simulink library "lib_all"  (Simulink 7.6)
settings: Solver=ode45  SolverMode=SingleTasking  StartTime=0.0  StopTime=10.0  RelTol=1e-3
values from neighbouring files (symbols this model uses):
  map_lib_03 = [6.8679 7.0159 7.4571 8.1849 9.1892 10.456 11.97 13.71 15.655 17.781 20.063 22.474 ... (803 elements, 73x11)]    [scr_simdata.mat]    (also in scr_simdata_all.mat)
  map_lib_06 = [2.5784 2.6727 2.9588 3.4453 4.1444 5.068 6.226 7.6242 9.2634 11.139 13.24 15.552 ... (803 elements, 73x11)]    [scr_simdata.mat]    (also in scr_simdata_all.mat)
  map_lib_09 = [0.0025784 0.0026727 0.0029588 0.0034453 0.0041444 0.005068 0.006226 0.0076242 0.0092634 0.011139 0.01324 0.015552 ... (803 elements, 73x11)]    [scr_simdata.mat]    (also in scr_simdata_all.mat)
  map_lib_10 = [2.74 2.75 2.76 2.77 2.78 2.79 2.8 2.81 2.82 2.83 2.84 2.85 ... (81 elements, 1x81)]    [scr_simdata.mat]    (also in scr_simdata_all.mat)
  map_lib_11 = [1.1983 1.2027 1.207 1.2113 1.2156 1.2198 1.2241 1.2283 1.2326 1.2368 1.241 1.2451 ... (81 elements, 1x81)]    [scr_simdata.mat]    (also in scr_simdata_all.mat)
  map_lib_12 = [2.74 2.75 2.76 2.77 2.78 2.79 2.8 2.81 2.82 2.83 2.84 2.85 ... (81 elements, 1x81)]    [scr_simdata.mat]    (also in scr_simdata_all.mat)
  map_lib_13 = [1.74 1.75 1.76 1.77 1.78 1.79 1.8 1.81 1.82 1.83 1.84 1.85 ... (81 elements, 1x81)]    [scr_simdata.mat]    (also in scr_simdata_all.mat)
  map_lib_16 = [0.00048828125 1.6159 1.8962 2.0821 2.2251 2.3426 2.4433 2.5318 2.611 2.683 2.749 2.8101 ... (1419 elements, 129x11)]    [scr_simdata.mat]    (also in scr_simdata_all.mat)
  map_mr2_01 = [0 800 1600 2400 3200 4000]    [scr_simdata.mat]    (also in scr_simdata_all.mat)
  map_mr2_02 = [1.32 1.32 1.33 1.34 1.36 1.38]    [scr_simdata.mat]    (also in scr_simdata_all.mat)
  map_mr2_03 = [0 800 1600 2400 3200 4000]    [scr_simdata.mat]    (also in scr_simdata_all.mat)
  map_mr2_04 = [1.32 1.32 1.33 1.34 1.36 1.38]    [scr_simdata.mat]    (also in scr_simdata_all.mat)
  map_mr2_05 = [0 800 1600 2400 3200 4000]    [scr_simdata.mat]    (also in scr_simdata_all.mat)
  map_mr2_06 = [1.32 1.32 1.33 1.34 1.36 1.38]    [scr_simdata.mat]    (also in scr_simdata_all.mat)
  Parameter1 = 6    [scr_simdata.mat]    (also in scr_simdata_all.mat)
  Parameter101 = 30    [scr_simdata.mat]    (also in scr_simdata_all.mat)
  Parameter102 = 12    [scr_simdata.mat]    (also in scr_simdata_all.mat)
  Parameter103 = -240    [scr_simdata.mat]    (also in scr_simdata_all.mat)
  Parameter108 = 48    [scr_simdata.mat]    (also in scr_simdata_all.mat)
  Parameter120 = 16    [scr_simdata.mat]    (also in scr_simdata_all.mat)
  Parameter121 = 87    [scr_simdata.mat]    (also in scr_simdata_all.mat)
  Parameter123 = 78    [scr_simdata.mat]    (also in scr_simdata_all.mat)
  Parameter124 = 60    [scr_simdata.mat]    (also in scr_simdata_all.mat)
  Parameter125 = -480    [scr_simdata.mat]    (also in scr_simdata_all.mat)
  Parameter130 = 96    [scr_simdata.mat]    (also in scr_simdata_all.mat)
  Parameter131 = 3    [scr_simdata.mat]    (also in scr_simdata_all.mat)
  Parameter144 = 3    [scr_simdata.mat]    (also in scr_simdata_all.mat)
  Parameter18 = 128    [scr_simdata.mat]    (also in scr_simdata_all.mat)
  Parameter19 = 16    [scr_simdata.mat]    (also in scr_simdata_all.mat)
  Parameter2 = 12    [scr_simdata.mat]    (also in scr_simdata_all.mat)
  Parameter20 = 7    [scr_simdata.mat]    (also in scr_simdata_all.mat)
  Parameter21 = 4    [scr_simdata.mat]    (also in scr_simdata_all.mat)
  Parameter3 = 18    [scr_simdata.mat]    (also in scr_simdata_all.mat)
  Parameter32 = 1    [scr_simdata.mat]    (also in scr_simdata_all.mat)
  Parameter34 = 24    [scr_simdata.mat]    (also in scr_simdata_all.mat)
  Parameter4 = 138    [scr_simdata.mat]    (also in scr_simdata_all.mat)
  Parameter42 = 48    [scr_simdata.mat]    (also in scr_simdata_all.mat)
  Parameter5 = 135    [scr_simdata.mat]    (also in scr_simdata_all.mat)
  Parameter55 = 0    [scr_simdata.mat]    (also in scr_simdata_all.mat)
  Parameter56 = 0    [scr_simdata.mat]    (also in scr_simdata_all.mat)
  Parameter57 = 1    [scr_simdata.mat]    (also in scr_simdata_all.mat)
  Parameter58 = 1    [scr_simdata.mat]    (also in scr_simdata_all.mat)
  Parameter59 = 2    [scr_simdata.mat]    (also in scr_simdata_all.mat)
  Parameter6 = 132    [scr_simdata.mat]    (also in scr_simdata_all.mat)
  Parameter60 = 2    [scr_simdata.mat]    (also in scr_simdata_all.mat)
  Parameter63 = 144    [scr_simdata.mat]    (also in scr_simdata_all.mat)
  Parameter7 = 126    [scr_simdata.mat]    (also in scr_simdata_all.mat)
  Parameter76 = 64    [scr_simdata.mat]    (also in scr_simdata_all.mat)
  Parameter77 = 135    [scr_simdata.mat]    (also in scr_simdata_all.mat)
  Parameter79 = 126    [scr_simdata.mat]    (also in scr_simdata_all.mat)
  Parameter80 = 108    [scr_simdata.mat]    (also in scr_simdata_all.mat)
  Parameter81 = 0    [scr_simdata.mat]    (also in scr_simdata_all.mat)
  Parameter86 = 0    [scr_simdata.mat]    (also in scr_simdata_all.mat)
  Parameter98 = 112    [scr_simdata.mat]    (also in scr_simdata_all.mat)
  Parameter99 = 39    [scr_simdata.mat]    (also in scr_simdata_all.mat)
undefined symbols (not defined in the file or in its folder): cylvolkp_hih_map2h, cylvolkp_map2h, pk1k_map2h
libraries: simulink x2
(part 14 of 36)

== system lib_all/SubSystem1920/SubSystem1928/SubSystem1941/SubSystem1947 ==
  Block5314 : Inport
  Block5315 : Inport  Port=2
  Block5316 : MultiPortSwitch  zeroidx=off  RndMeth=Zero  SaturateOnIntegerOverflow=on  InputSameDT=on
  SubSystem1948 : Subsystem  TreatAsAtomicUnit=on  mask(***ATC.DSRVEC***){bd_version=1.01.A, r0=DSM18, r1=-1, r2=, r3=, r4=, r5=, r6=, r7=, r8=, r9=, c0=, c1=, c2=, c3=, c4=, c5=, c6=, c7=, c8=, c9=}
      mask prompts: bd_version "Version"
      mask help: これはAutoCからのブロックです．編集禁止．
      settings: OpenFcn=warndlg('変更禁止!');
      mask Help: web(ui_blockhelpmng(gcb));
      mask Display: disp('ATC.DSRVEC')
  SubSystem1949 : Subsystem  TreatAsAtomicUnit=on  mask(***ATC.DSRVEC***){bd_version=1.01.A, r0=DSM8, r1=-1, r2=, r3=, r4=, r5=, r6=, r7=, r8=, r9=, c0=, c1=, c2=, c3=, c4=, c5=, c6=, c7=, c8=, c9=}
      mask prompts: bd_version "Version"
      mask help: これはAutoCからのブロックです．編集禁止．
      settings: OpenFcn=warndlg('変更禁止!');
      mask Help: web(ui_blockhelpmng(gcb));
      mask Display: disp('ATC.DSRVEC')
  SubSystem1950 : Subsystem  TreatAsAtomicUnit=on  mask(***ATC.DSRVEC***){bd_version=1.01.A, r0=DSM11, r1=-1, r2=, r3=, r4=, r5=, r6=, r7=, r8=, r9=, c0=, c1=, c2=, c3=, c4=, c5=, c6=, c7=, c8=, c9=}
      mask prompts: bd_version "Version"
      mask help: これはAutoCからのブロックです．編集禁止．
      settings: OpenFcn=warndlg('変更禁止!');
      mask Help: web(ui_blockhelpmng(gcb));
      mask Display: disp('ATC.DSRVEC')
  Block5317 : Outport
  wires:
  Block5315/1 -> SubSystem1950/1(element), SubSystem1949/1(element), SubSystem1948/1(element)
  SubSystem1950/1(Output) -> Block5316/4
  SubSystem1949/1(Output) -> Block5316/3
  SubSystem1948/1(Output) -> Block5316/2
  Block5316/1 -> Block5317/1
  Block5314/1 -> Block5316/1

== system lib_all/SubSystem1920/SubSystem1928/SubSystem1951/SubSystem1952 ==
  note: ***編集禁止***
  element : Inport
  Inside_DSR : DataStoreRead  DataStoreName=DSM2
  Selector : Selector  IndexOptions=Index vector (port)  Indices=[1 3]  OutputSizes=1
  Output : Outport
  wires:
  Inside_DSR/1 -> Selector/1
  element/1 -> Selector/2
  Selector/1 -> Output/1

== system lib_all/SubSystem1920/SubSystem1928/SubSystem1951/SubSystem1953 ==
  Block5325 : Inport
  Block5326 : Inport  Port=2
  Block5327 : MultiPortSwitch  zeroidx=off  RndMeth=Zero  SaturateOnIntegerOverflow=on  InputSameDT=on
  SubSystem1954 : Subsystem  TreatAsAtomicUnit=on  mask(***ATC.DSRVEC***){bd_version=1.01.A, r0=DSM18, r1=-1, r2=, r3=, r4=, r5=, r6=, r7=, r8=, r9=, c0=, c1=, c2=, c3=, c4=, c5=, c6=, c7=, c8=, c9=}
      mask prompts: bd_version "Version"
      mask help: これはAutoCからのブロックです．編集禁止．
      settings: OpenFcn=warndlg('変更禁止!');
      mask Help: web(ui_blockhelpmng(gcb));
      mask Display: disp('ATC.DSRVEC')
  SubSystem1955 : Subsystem  TreatAsAtomicUnit=on  mask(***ATC.DSRVEC***){bd_version=1.01.A, r0=DSM8, r1=-1, r2=, r3=, r4=, r5=, r6=, r7=, r8=, r9=, c0=, c1=, c2=, c3=, c4=, c5=, c6=, c7=, c8=, c9=}
      mask prompts: bd_version "Version"
      mask help: これはAutoCからのブロックです．編集禁止．
      settings: OpenFcn=warndlg('変更禁止!');
      mask Help: web(ui_blockhelpmng(gcb));
      mask Display: disp('ATC.DSRVEC')
  SubSystem1956 : Subsystem  TreatAsAtomicUnit=on  mask(***ATC.DSRVEC***){bd_version=1.01.A, r0=DSM11, r1=-1, r2=, r3=, r4=, r5=, r6=, r7=, r8=, r9=, c0=, c1=, c2=, c3=, c4=, c5=, c6=, c7=, c8=, c9=}
      mask prompts: bd_version "Version"
      mask help: これはAutoCからのブロックです．編集禁止．
      settings: OpenFcn=warndlg('変更禁止!');
      mask Help: web(ui_blockhelpmng(gcb));
      mask Display: disp('ATC.DSRVEC')
  Block5328 : Outport
  wires:
  Block5326/1 -> SubSystem1956/1(element), SubSystem1955/1(element), SubSystem1954/1(element)
  SubSystem1956/1(Output) -> Block5327/4
  SubSystem1955/1(Output) -> Block5327/3
  SubSystem1954/1(Output) -> Block5327/2
  Block5327/1 -> Block5328/1
  Block5325/1 -> Block5327/1

== system lib_all/SubSystem1920/SubSystem1928/SubSystem1951/SubSystem1957 ==
  Block5329 : Inport
  Block5330 : Inport  Port=2
  Block5331 : MultiPortSwitch  zeroidx=off  RndMeth=Zero  SaturateOnIntegerOverflow=on  InputSameDT=on
  SubSystem1958 : Subsystem  TreatAsAtomicUnit=on  mask(***ATC.DSRVEC***){bd_version=1.01.A, r0=DSM18, r1=-1, r2=, r3=, r4=, r5=, r6=, r7=, r8=, r9=, c0=, c1=, c2=, c3=, c4=, c5=, c6=, c7=, c8=, c9=}
      mask prompts: bd_version "Version"
      mask help: これはAutoCからのブロックです．編集禁止．
      settings: OpenFcn=warndlg('変更禁止!');
      mask Help: web(ui_blockhelpmng(gcb));
      mask Display: disp('ATC.DSRVEC')
  SubSystem1959 : Subsystem  TreatAsAtomicUnit=on  mask(***ATC.DSRVEC***){bd_version=1.01.A, r0=DSM8, r1=-1, r2=, r3=, r4=, r5=, r6=, r7=, r8=, r9=, c0=, c1=, c2=, c3=, c4=, c5=, c6=, c7=, c8=, c9=}
      mask prompts: bd_version "Version"
      mask help: これはAutoCからのブロックです．編集禁止．
      settings: OpenFcn=warndlg('変更禁止!');
      mask Help: web(ui_blockhelpmng(gcb));
      mask Display: disp('ATC.DSRVEC')
  SubSystem1960 : Subsystem  TreatAsAtomicUnit=on  mask(***ATC.DSRVEC***){bd_version=1.01.A, r0=DSM11, r1=-1, r2=, r3=, r4=, r5=, r6=, r7=, r8=, r9=, c0=, c1=, c2=, c3=, c4=, c5=, c6=, c7=, c8=, c9=}
      mask prompts: bd_version "Version"
      mask help: これはAutoCからのブロックです．編集禁止．
      settings: OpenFcn=warndlg('変更禁止!');
      mask Help: web(ui_blockhelpmng(gcb));
      mask Display: disp('ATC.DSRVEC')
  Block5332 : Outport
  wires:
  Block5330/1 -> SubSystem1960/1(element), SubSystem1959/1(element), SubSystem1958/1(element)
  SubSystem1960/1(Output) -> Block5331/4
  SubSystem1959/1(Output) -> Block5331/3
  SubSystem1958/1(Output) -> Block5331/2
  Block5331/1 -> Block5332/1
  Block5329/1 -> Block5331/1

== system lib_all/SubSystem347/SubSystem349/SubSystem352/SubSystem353 ==
  note: Text58
  Block776 : Inport
  Block777 : Inport  Port=2
  Block778 : Inport  Port=3
  Block779 : Inport  Port=4
  Block780 : Constant  Value=0  OutDataTypeStr=double  SampleTime=-1
  Block781 : Constant  OutDataTypeStr=double  SampleTime=-1
  Block782 : Constant  Value=0  OutDataTypeStr=double  SampleTime=-1
  Block783 : Gain  Gain=1/5  SaturateOnIntegerOverflow=on  OutDataTypeStr=Inherit: Same as input  ParamDataTypeStr=Inherit: Same as input
      settings: AttributesFormatString=Attribute175
  Block784 : Product  OutDataTypeStr=double  SaturateOnIntegerOverflow=on  RndMeth=Zero  InputSameDT=on
  Block785 : Sum  Inputs=-+  OutDataTypeStr=double  RndMeth=Zero  SaturateOnIntegerOverflow=on  InputSameDT=on
  Block786 : Sum  OutDataTypeStr=double  RndMeth=Zero  SaturateOnIntegerOverflow=on  InputSameDT=on
  Block787 : Sum  OutDataTypeStr=double  RndMeth=Zero  SaturateOnIntegerOverflow=on  InputSameDT=on
  Block788 : Switch  Threshold=0.5  RndMeth=Zero  SaturateOnIntegerOverflow=on  InputSameDT=on
  SubSystem355 : Subsystem
  SubSystem356 : Subsystem
  SubSystem362 : Subsystem
  Block897 : Outport
  wires:
  Block787/1 -> SubSystem362/3(Block818), SubSystem356/2(Block790)
  Block776/1 -> Block787/1
  Block783/1 -> Block786/2
  Block784/1 -> Block783/1
  Block781/1 -> Block787/2
  Block780/1 -> Block788/1
  Block788/1 -> Block897/1
  SubSystem355/1(Out1) -> Block788/2
  Block782/1 -> SubSystem355/2(In2)
  Block778/1 -> SubSystem356/3(Block791)
  Block786/1 -> SubSystem355/1(In1), Block788/3
  SubSystem356/2(Block815) -> Block784/2
  Block785/1 -> Block784/1  "SigLabel19"
  SubSystem362/2(Block896) -> Block785/2
  SubSystem362/1(Block895) -> Block785/1, Block786/1
  Block779/1 -> SubSystem362/2(Block817)
  SubSystem356/1(Block814) -> SubSystem362/1(Block816)
  Block777/1 -> SubSystem356/1(Block789)

== system lib_all/SubSystem347/SubSystem349/SubSystem352/SubSystem406 ==
  note: Text64
  Block898 : Inport
  Block899 : Inport  Port=2
  Block900 : Inport  Port=3
  Block901 : Inport  Port=4
  Block902 : Constant  Value=0  OutDataTypeStr=double  SampleTime=-1
  Block903 : Constant  OutDataTypeStr=double  SampleTime=-1
  Block904 : Constant  Value=0  OutDataTypeStr=double  SampleTime=-1
  Block905 : Gain  Gain=1/5  SaturateOnIntegerOverflow=on  OutDataTypeStr=Inherit: Same as input  ParamDataTypeStr=Inherit: Same as input
      settings: AttributesFormatString=Attribute194
  Block906 : Product  OutDataTypeStr=double  SaturateOnIntegerOverflow=on  RndMeth=Zero  InputSameDT=on
  Block907 : Sum  Inputs=-+  OutDataTypeStr=double  RndMeth=Zero  SaturateOnIntegerOverflow=on  InputSameDT=on
  Block908 : Sum  OutDataTypeStr=double  RndMeth=Zero  SaturateOnIntegerOverflow=on  InputSameDT=on
  Block909 : Sum  OutDataTypeStr=double  RndMeth=Zero  SaturateOnIntegerOverflow=on  InputSameDT=on
  Block910 : Switch  Threshold=0.5  RndMeth=Zero  SaturateOnIntegerOverflow=on  InputSameDT=on
  SubSystem408 : Subsystem
  SubSystem409 : Subsystem
  SubSystem415 : Subsystem
  Block1019 : Outport
  wires:
  Block909/1 -> SubSystem415/3(Block940), SubSystem409/2(Block912)
  Block898/1 -> Block909/1
  Block905/1 -> Block908/2
  Block906/1 -> Block905/1
  Block903/1 -> Block909/2
  Block902/1 -> Block910/1
  Block910/1 -> Block1019/1
  SubSystem408/1(Out1) -> Block910/2
  Block904/1 -> SubSystem408/2(In2)
  Block900/1 -> SubSystem409/3(Block913)
  Block908/1 -> SubSystem408/1(In1), Block910/3
  SubSystem409/2(Block937) -> Block906/2
  Block907/1 -> Block906/1  "SigLabel22"
  SubSystem415/2(Block1018) -> Block907/2
  SubSystem415/1(Block1017) -> Block907/1, Block908/1
  Block901/1 -> SubSystem415/2(Block939)
  SubSystem409/1(Block936) -> SubSystem415/1(Block938)
  Block899/1 -> SubSystem409/1(Block911)

== system lib_all/SubSystem347/SubSystem349/SubSystem352/SubSystem459 ==
  note: Text65
  Block1020 : Inport
  Block1021 : Inport  Port=2
  Block1022 : Inport  Port=3
  Block1023 : SwitchCase  CaseConditions={1 2 3}
  Block1024 : Terminator
  SubSystem460 : Subsystem
      settings: RTWSystemCode=Inline
  SubSystem462 : Subsystem
      settings: RTWSystemCode=Inline
  SubSystem464 : Subsystem
      settings: RTWSystemCode=Inline
  wires:
  Block1021/1 -> Block1023/1
  Block1020/1 -> SubSystem460/1(Block1025), SubSystem462/1(Block1028), SubSystem464/1(Block1031)
  Block1022/1 -> SubSystem460/2(Block1026), SubSystem462/2(Block1029), SubSystem464/2(Block1032)
  Block1023/1 -> SubSystem460/Action
  Block1023/2 -> SubSystem462/Action
  Block1023/4 -> Block1024/1
  Block1023/3 -> SubSystem464/Action

== system lib_all/SubSystem347/SubSystem349/SubSystem352/SubSystem466 ==
  note: Text66
  Block1034 : Inport
  Block1035 : Inport  Port=2
  Block1036 : Inport  Port=3
  Block1037 : SwitchCase  CaseConditions={1 2 3}
  Block1038 : Terminator
  SubSystem467 : Subsystem
      settings: RTWSystemCode=Inline
  SubSystem469 : Subsystem
      settings: RTWSystemCode=Inline
  SubSystem471 : Subsystem
      settings: RTWSystemCode=Inline
  wires:
  Block1037/2 -> SubSystem469/Action
  Block1037/1 -> SubSystem467/Action
  Block1036/1 -> SubSystem469/2(Block1043), SubSystem471/2(Block1046), SubSystem467/2(Block1040)
  Block1034/1 -> SubSystem469/1(Block1042), SubSystem471/1(Block1045), SubSystem467/1(Block1039)
  Block1035/1 -> Block1037/1
  Block1037/3 -> SubSystem471/Action
  Block1037/4 -> Block1038/1

== system lib_all/SubSystem347/SubSystem349/SubSystem352/SubSystem473 ==
  Block1048 : Inport
  Block1049 : Constant  OutDataTypeStr=double  SampleTime=-1
  Block1050 : Constant  OutDataTypeStr=double  SampleTime=-1
  Block1051 : Sum  OutDataTypeStr=double  RndMeth=Zero  SaturateOnIntegerOverflow=on  InputSameDT=on
  Block1052 : Sum  OutDataTypeStr=double  RndMeth=Zero  SaturateOnIntegerOverflow=on  InputSameDT=on
  SubSystem474 : Subsystem  TreatAsAtomicUnit=on  mask(***ATC.DSRVEC***){bd_version=1.01.A, r0=DSM386, r1=-1, r2=, r3=, r4=, r5=, r6=, r7=, r8=, r9=, c0=, c1=, c2=, c3=, c4=, c5=, c6=, c7=, c8=, c9=}
      mask prompts: bd_version "Version"
      mask help: これはAutoCからのブロックです．編集禁止．
      settings: OpenFcn=warndlg('変更禁止!');
      mask Help: web(ui_blockhelpmng(gcb));
      mask Display: disp('ATC.DSRVEC')
  SubSystem475 : Subsystem  TreatAsAtomicUnit=on  mask(***ATC.DSWVEC***){bd_version=1.01.A, r0=DSM386, r1=-1, r2=, r3=, r4=, r5=, r6=, r7=, r8=, r9=, c0=, c1=, c2=, c3=, c4=, c5=, c6=, c7=, c8=, c9=}
      mask prompts: bd_version "Version"
      mask help: これはAutoCからのブロックです．編集禁止．
      settings: OpenFcn=warndlg('変更禁止!');
      mask Help: web(ui_blockhelpmng(gcb));
      mask Display: disp('ATC.DSRVEC')
  wires:
  Block1050/1 -> Block1052/1
  Block1049/1 -> Block1051/2
  Block1048/1 -> Block1051/1
  SubSystem474/1(Output) -> Block1052/2
  Block1051/1 -> SubSystem474/1(element), SubSystem475/2(element)
  Block1052/1 -> SubSystem475/1(input)

== system lib_all/SubSystem347/SubSystem477/SubSystem479/SubSystem480 ==
  note: ***編集禁止***
  N : Inport
  For_loop : Stateflow chart  TreatAsAtomicUnit=on
  action : Outport
  i : Outport  Port=2
  wires:
  For_loop/2(function_call) -> action/1
  N/1 -> For_loop/1(N_Loop)
  For_loop/1(idx) -> i/1

== system lib_all/SubSystem347/SubSystem477/SubSystem479/SubSystem482 ==
  Block1075 : Inport
  Block1076 : Inport  Port=2
  Block1077 : Inport  Port=3
  Block1078 : Constant  Value=Parameter20  OutDataTypeStr=double  SampleTime=-1
  Block1079 : Constant  Value=2  OutDataTypeStr=double  SampleTime=-1
  Block1080 : Constant  Value=0  OutDataTypeStr=double  SampleTime=-1
  Block1081 : Math  Operator=pow  SaturateOnIntegerOverflow=on
  Block1082 : Product  Inputs=*/  OutDataTypeStr=double  SaturateOnIntegerOverflow=on  RndMeth=Zero  InputSameDT=on
  Block1083 : Product  Inputs=*/  OutDataTypeStr=double  SaturateOnIntegerOverflow=on  RndMeth=Zero  InputSameDT=on
  Block1084 : Sum  Inputs=-+  OutDataTypeStr=double  RndMeth=Zero  SaturateOnIntegerOverflow=on  InputSameDT=on
  SubSystem483 : Subsystem
  SubSystem484 : Subsystem  Priority=1  TreatAsAtomicUnit=on  mask(***ATC.DSWVEC***){bd_version=1.01.A, r0=DSM383, r1=-1, r2=, r3=, r4=, r5=, r6=, r7=, r8=, r9=, c0=, c1=, c2=, c3=, c4=, c5=, c6=, c7=, c8=, c9=}
      mask prompts: bd_version "Version"
      mask help: これはAutoCからのブロックです．編集禁止．
      settings: AttributesFormatString=Attribute225  OpenFcn=warndlg('変更禁止!');
      mask Help: web(ui_blockhelpmng(gcb));
      mask Display: disp('ATC.DSRVEC')
  SubSystem485 : Subsystem  Priority=2  TreatAsAtomicUnit=on  mask(***ATC.DSWVEC***){bd_version=1.01.A, r0=DSM385, r1=-1, r2=, r3=, r4=, r5=, r6=, r7=, r8=, r9=, c0=, c1=, c2=, c3=, c4=, c5=, c6=, c7=, c8=, c9=}
      mask prompts: bd_version "Version"
      mask help: これはAutoCからのブロックです．編集禁止．
      settings: AttributesFormatString=Attribute226  OpenFcn=warndlg('変更禁止!');
      mask Help: web(ui_blockhelpmng(gcb));
      mask Display: disp('ATC.DSRVEC')
  SubSystem486 : Subsystem  Priority=3  TreatAsAtomicUnit=on  mask(***ATC.DSWVEC***){bd_version=1.01.A, r0=DSM387, r1=-1, r2=, r3=, r4=, r5=, r6=, r7=, r8=, r9=, c0=, c1=, c2=, c3=, c4=, c5=, c6=, c7=, c8=, c9=}
      mask prompts: bd_version "Version"
      mask help: これはAutoCからのブロックです．編集禁止．
      settings: AttributesFormatString=Attribute227  OpenFcn=warndlg('変更禁止!');
      mask Help: web(ui_blockhelpmng(gcb));
      mask Display: disp('ATC.DSRVEC')
  SubSystem487 : Subsystem  Priority=4
      settings: AttributesFormatString=Attribute228
  wires:
  Block1079/1 -> Block1081/1
  Block1078/1 -> Block1081/2
  Block1082/1 -> SubSystem484/1(input), Block1084/2
  Block1083/1 -> SubSystem485/1(input), Block1084/1
  Block1075/1 -> Block1082/1
  Block1077/1 -> SubSystem484/2(element), SubSystem486/2(element), SubSystem485/2(element)
  Block1076/1 -> Block1083/1
  Block1084/1 -> SubSystem486/1(input), SubSystem483/1(In1)
  Block1080/1 -> SubSystem483/2(In2)
  SubSystem483/1(Out1) -> SubSystem487/Enable
  Block1081/1 -> Block1082/2, Block1083/2

== system lib_all/SubSystem347/SubSystem477/SubSystem479/SubSystem492 ==
  Block1095 : Inport
  Block1096 : Inport  Port=2
  Block1097 : TriggerPort  TriggerType=function-call
  Block1098 : Constant  OutDataTypeStr=double  SampleTime=-1
  Block1099 : Sum  OutDataTypeStr=double  RndMeth=Zero  SaturateOnIntegerOverflow=on  InputSameDT=on
  Block1100 : Sum  OutDataTypeStr=double  RndMeth=Zero  SaturateOnIntegerOverflow=on  InputSameDT=on
  Block1101 : Switch  Threshold=0.5  RndMeth=Zero  SaturateOnIntegerOverflow=on  InputSameDT=on
  Block1102 : Switch  Threshold=0.5  RndMeth=Zero  SaturateOnIntegerOverflow=on  InputSameDT=on
  Block1103 : UnitDelay  SampleTime=-1
  Block1104 : UnitDelay  SampleTime=-1
  SubSystem493 : Subsystem
  SubSystem494 : Subsystem
  SubSystem498 : Subsystem
  Block1113 : Outport
  Block1114 : Outport  Port=2
  wires:
  Block1095/1 -> SubSystem494/2(Block1106), SubSystem498/2(Block1110), SubSystem493/1(In1)
  Block1098/1 -> SubSystem493/2(In2)
  SubSystem493/1(Out1) -> Block1101/2, Block1102/2
  Block1101/1 -> Block1113/1, Block1103/1
  Block1096/1 -> SubSystem494/1(Block1105), SubSystem498/1(Block1109)
  Block1099/1 -> Block1101/3
  SubSystem494/1(Block1108) -> Block1099/1, Block1101/1
  Block1103/1 -> Block1099/2
  Block1102/1 -> Block1114/1, Block1104/1
  Block1100/1 -> Block1102/3
  SubSystem498/1(Block1112) -> Block1100/1, Block1102/1
  Block1104/1 -> Block1100/2

== system lib_all/SubSystem347/SubSystem503/SubSystem506/SubSystem507 ==
  note: ***編集禁止***
  N : Inport
  For_loop : Stateflow chart  TreatAsAtomicUnit=on
  action : Outport
  i : Outport  Port=2
  wires:
  For_loop/2(function_call) -> action/1
  N/1 -> For_loop/1(N_Loop)
  For_loop/1(idx) -> i/1

== system lib_all/SubSystem347/SubSystem503/SubSystem506/SubSystem509 ==
  note: ***編集禁止***
  N : Inport
  For_loop : Stateflow chart  TreatAsAtomicUnit=on
  action : Outport
  i : Outport  Port=2
  wires:
  For_loop/2(function_call) -> action/1
  N/1 -> For_loop/1(N_Loop)
  For_loop/1(idx) -> i/1

== system lib_all/SubSystem347/SubSystem503/SubSystem506/SubSystem511 ==
  Block1127 : Inport
  Block1128 : TriggerPort  TriggerType=function-call
  Block1129 : Constant  OutDataTypeStr=double  SampleTime=-1
  Block1130 : Product  Inputs=*/  OutDataTypeStr=double  SaturateOnIntegerOverflow=on  RndMeth=Zero  InputSameDT=on
  SubSystem512 : Subsystem  TreatAsAtomicUnit=on  mask(***ATC.DSWVEC***){bd_version=1.01.A, r0=DSM87, r1=-1, r2=, r3=, r4=, r5=, r6=, r7=, r8=, r9=, c0=, c1=, c2=, c3=, c4=, c5=, c6=, c7=, c8=, c9=}
      mask prompts: bd_version "Version"
      mask help: これはAutoCからのブロックです．編集禁止．
      settings: OpenFcn=warndlg('変更禁止!');
      mask Help: web(ui_blockhelpmng(gcb));
      mask Display: disp('ATC.DSRVEC')
  SubSystem513 : Subsystem  TreatAsAtomicUnit=on  mask(***ATC.DSRVEC***){bd_version=1.01.A, r0=DSM387, r1=-1, r2=, r3=, r4=, r5=, r6=, r7=, r8=, r9=, c0=, c1=, c2=, c3=, c4=, c5=, c6=, c7=, c8=, c9=}
      mask prompts: bd_version "Version"
      mask help: これはAutoCからのブロックです．編集禁止．
      settings: OpenFcn=warndlg('変更禁止!');
      mask Help: web(ui_blockhelpmng(gcb));
      mask Display: disp('ATC.DSRVEC')
  SubSystem514 : Subsystem  TreatAsAtomicUnit=on  mask(***ATC.DSRVEC***){bd_version=1.01.A, r0=DSM387, r1=-1, r2=, r3=, r4=, r5=, r6=, r7=, r8=, r9=, c0=, c1=, c2=, c3=, c4=, c5=, c6=, c7=, c8=, c9=}
      mask prompts: bd_version "Version"
      mask help: これはAutoCからのブロックです．編集禁止．
      settings: OpenFcn=warndlg('変更禁止!');
      mask Help: web(ui_blockhelpmng(gcb));
      mask Display: disp('ATC.DSRVEC')
  wires:
  Block1129/1 -> SubSystem514/1(element)
  Block1127/1 -> SubSystem512/2(element), SubSystem513/1(element)
  SubSystem514/1(Output) -> Block1130/1
  SubSystem513/1(Output) -> Block1130/2
  Block1130/1 -> SubSystem512/1(input)

== system lib_all/SubSystem347/SubSystem503/SubSystem506/SubSystem515 ==
  Block1131 : Inport
  Block1132 : TriggerPort  TriggerType=function-call
  Block1133 : Constant  Value=0  OutDataTypeStr=double  SampleTime=-1
  SubSystem516 : Subsystem  TreatAsAtomicUnit=on  mask(***ATC.DSWVEC***){bd_version=1.01.A, r0=DSM386, r1=-1, r2=, r3=, r4=, r5=, r6=, r7=, r8=, r9=, c0=, c1=, c2=, c3=, c4=, c5=, c6=, c7=, c8=, c9=}
      mask prompts: bd_version "Version"
      mask help: これはAutoCからのブロックです．編集禁止．
      settings: OpenFcn=warndlg('変更禁止!');
      mask Help: web(ui_blockhelpmng(gcb));
      mask Display: disp('ATC.DSRVEC')
  wires:
  Block1131/1 -> SubSystem516/2(element)
  Block1133/1 -> SubSystem516/1(input)

== system lib_all/SubSystem347/SubSystem503/SubSystem506/SubSystem517 ==
  Block1134 : Constant  Value=0  OutDataTypeStr=double  SampleTime=-1
  Block1135 : DataStoreWrite  DataStoreName=DSM365
  wires:
  Block1134/1 -> Block1135/1

== system lib_all/SubSystem518/SubSystem523/SubSystem524/SubSystem526 ==
  note: ***編集禁止***
  In1 : Inport
  In2 : Inport  Port=2
  LO1 : Logic
  RO1 : RelationalOperator  Operator=<  InputSameDT=on
  RO2 : RelationalOperator  Operator=~=  InputSameDT=on
  single1 : DataTypeConversion  OutDataTypeStr=single  SaturateOnIntegerOverflow=on  RndMeth=Zero
  single2 : DataTypeConversion  OutDataTypeStr=single  SaturateOnIntegerOverflow=on  RndMeth=Zero
  Out1 : Outport
  wires:
  LO1/1 -> Out1/1
  RO2/1 -> LO1/2
  RO1/1 -> LO1/1
  single2/1 -> RO2/2, RO1/2
  single1/1 -> RO2/1, RO1/1
  In1/1 -> single1/1
  In2/1 -> single2/1

== system lib_all/SubSystem518/SubSystem523/SubSystem524/SubSystem527 ==
  note: Text76
  note: Text75
  Block1176 : Inport
  Block1177 : Inport  Port=2
  Block1178 : Inport  Port=3
  Block1179 : Constant  Value=720  OutDataTypeStr=double  SampleTime=-1
  Block1180 : Constant  Value=0  OutDataTypeStr=double  SampleTime=-1
  Block1181 : Constant  Value=0  OutDataTypeStr=double  SampleTime=-1
  Block1182 : Constant  Value=5  OutDataTypeStr=double  SampleTime=-1
  Block1183 : DataStoreRead  DataStoreName=DSM50
  Block1184 : DataStoreRead  DataStoreName=DSM35
  Block1185 : Gain  Gain=5  SaturateOnIntegerOverflow=on  OutDataTypeStr=Inherit: Same as input  ParamDataTypeStr=Inherit: Same as input
      settings: AttributesFormatString=Attribute265
  Block1186 : Gain  Gain=5  SaturateOnIntegerOverflow=on  OutDataTypeStr=Inherit: Same as input  ParamDataTypeStr=Inherit: Same as input
      settings: AttributesFormatString=Attribute266
  Block1187 : Gain  Gain=1/5  SaturateOnIntegerOverflow=on  OutDataTypeStr=Inherit: Same as input  ParamDataTypeStr=Inherit: Same as input
      settings: AttributesFormatString=Attribute267
  Block1188 : Product  Inputs=*/  OutDataTypeStr=double  SaturateOnIntegerOverflow=on  RndMeth=Zero  InputSameDT=on
  Block1189 : Rounding
  Block1190 : Rounding
  Block1191 : Sum  Inputs=+-+-  OutDataTypeStr=double  RndMeth=Zero  SaturateOnIntegerOverflow=on  InputSameDT=on
  Block1192 : Sum  Inputs=-+  OutDataTypeStr=double  RndMeth=Zero  SaturateOnIntegerOverflow=on  InputSameDT=on
  SubSystem528 : Subsystem  TreatAsAtomicUnit=on  mask(***ATC.DIVISION***){bd_version=1.01.A, r0=チェック有り, r1=, r2=, r3=, r4=, r5=, r6=, r7=, r8=, r9=, c0=, c1=, c2=, c3=, c4=, c5=, c6=, c7=, c8=, c9=}
      mask prompts: bd_version "Version"
      mask help: これはAutoCからのブロックです．編集禁止．
      settings: OpenFcn=warndlg('変更禁止!');
      mask Help: web(ui_blockhelpmng(gcb));
  SubSystem529 : Subsystem  TreatAsAtomicUnit=on  mask(***ATC.DSRVEC***){bd_version=1.01.A, r0=DSM95, r1=-1, r2=, r3=, r4=, r5=, r6=, r7=, r8=, r9=, c0=, c1=, c2=, c3=, c4=, c5=, c6=, c7=, c8=, c9=}
      mask prompts: bd_version "Version"
      mask help: これはAutoCからのブロックです．編集禁止．
      settings: OpenFcn=warndlg('変更禁止!');
      mask Help: web(ui_blockhelpmng(gcb));
      mask Display: disp('ATC.DSRVEC')
  SubSystem530 : Subsystem
      settings: RTWSystemCode=Inline
  Block1201 : Outport
  Block1202 : Outport  Port=2
  wires:
  Block1184/1 -> SubSystem528/2(div)
  Block1192/1 -> Block1202/1
  Block1185/1 -> Block1192/1
  Block1189/1 -> Block1185/1
  Block1188/1 -> Block1189/1
  Block1182/1 -> Block1188/2
  Block1177/1 -> SubSystem529/1(element)
  Block1181/1 -> SubSystem530/3(Block1195)
  Block1191/1 -> SubSystem530/2(Block1194)  "SigLabel27"
  Block1179/1 -> SubSystem530/1(Block1193)
  Block1178/1 -> Block1191/4
  SubSystem530/1(Block1200) -> Block1188/1, Block1192/2, Block1187/1
  Block1176/1 -> Block1186/1
  SubSystem528/1(Out) -> Block1191/3  "SigLabel26"
  Block1180/1 -> SubSystem528/3(init)
  SubSystem529/1(Output) -> SubSystem528/1(mult)
  Block1183/1 -> Block1191/2
  Block1186/1 -> Block1191/1
  Block1187/1 -> Block1190/1
  Block1190/1 -> Block1201/1

== system lib_all/SubSystem518/SubSystem523/SubSystem524/SubSystem533 ==
  note: Text79
  note: Text78
  note: Text77
  Block1203 : Inport
  Block1204 : Inport  Port=2
  Block1205 : Inport  Port=3
  Block1206 : Constant  Value=0  OutDataTypeStr=double  SampleTime=-1
  Block1207 : Constant  OutDataTypeStr=double  SampleTime=-1
  Block1208 : Constant  OutDataTypeStr=double  SampleTime=-1
  Block1209 : Constant  Value=0  OutDataTypeStr=double  SampleTime=-1
  Block1210 : Constant  Value=2  OutDataTypeStr=double  SampleTime=-1
  Block1211 : Constant  OutDataTypeStr=double  SampleTime=-1
  Block1212 : Constant  Value=143  OutDataTypeStr=double  SampleTime=-1
  Block1213 : Demux  Outputs=2
  Block1214 : Logic  Operator=NOT
  Block1215 : Mux  Inputs=2
  Block1216 : Mux  Inputs=2
  Block1217 : Sum  OutDataTypeStr=double  RndMeth=Zero  SaturateOnIntegerOverflow=on  InputSameDT=on
  Block1218 : Sum  OutDataTypeStr=double  RndMeth=Zero  SaturateOnIntegerOverflow=on  InputSameDT=on
  Block{1219..1222} (4 alike) : Switch  Threshold=0.5  RndMeth=Zero  SaturateOnIntegerOverflow=on  InputSameDT=on
  SubSystem534 : Subsystem
  SubSystem535 : Subsystem
  SubSystem536 : Subsystem
  SubSystem537 : Subsystem
  SubSystem538 : Subsystem  TreatAsAtomicUnit=on
  SubSystem547 : Subsystem  TreatAsAtomicUnit=on
  SubSystem557 : Subsystem  TreatAsAtomicUnit=on
  SubSystem567 : Subsystem  TreatAsAtomicUnit=on
  Block1282 : Outport
  Block1283 : Outport  Port=2
  wires:
  Block1205/1 -> SubSystem567/2(Block1268), SubSystem557/2(Block1253), SubSystem547/2(Block1238), SubSystem538/2(Block1224)
  Block1222/1 -> Block1220/3
  SubSystem567/1(Block1281) -> Block1222/3
  SubSystem557/1(Block1266) -> Block1222/1
  Block1214/1 -> SubSystem567/Enable
  SubSystem537/1(Out1) -> Block1222/2, SubSystem557/Enable, Block1214/1
  Block1210/1 -> SubSystem537/2(In2)
  Block1215/1 -> Block1221/1
  Block1217/1 -> Block1215/2
  Block1216/1 -> Block1221/3
  Block1209/1 -> Block1216/2
  Block1208/1 -> Block1217/1
  SubSystem534/1(Out1) -> Block1221/2
  Block1219/1 -> Block1213/1
  Block1213/2 -> Block1283/1
  Block1213/1 -> Block1282/1
  Block1218/1 -> SubSystem567/1(Block1267), SubSystem557/1(Block1252), SubSystem547/1(Block1237), SubSystem538/1(Block1223)
  Block1220/1 -> Block1219/3
  SubSystem547/1(Block1251) -> Block1220/1
  SubSystem536/1(Out1) -> SubSystem547/Enable, Block1220/2
  Block1207/1 -> SubSystem536/2(In2)
  SubSystem538/1(Block1236) -> Block1219/1
  SubSystem535/1(Out1) -> SubSystem538/Enable, Block1219/2
  Block1206/1 -> SubSystem535/2(In2)
  Block1204/1 -> SubSystem537/1(In1), SubSystem536/1(In1), SubSystem535/1(In1)
  Block1212/1 -> Block1216/1, SubSystem534/2(In2)
  Block1203/1 -> Block1215/1, Block1217/2, SubSystem534/1(In1)
  Block1211/1 -> Block1218/1
  Block1221/1 -> Block1218/2

== system lib_all/SubSystem518/SubSystem523/SubSystem577/SubSystem579 ==
  note: ***編集禁止***
  In1 : Inport
  In2 : Inport  Port=2
  LO1 : Logic
  RO1 : RelationalOperator  Operator=<  InputSameDT=on
  RO2 : RelationalOperator  Operator=~=  InputSameDT=on
  single1 : DataTypeConversion  OutDataTypeStr=single  SaturateOnIntegerOverflow=on  RndMeth=Zero
  single2 : DataTypeConversion  OutDataTypeStr=single  SaturateOnIntegerOverflow=on  RndMeth=Zero
  Out1 : Outport
  wires:
  LO1/1 -> Out1/1
  RO2/1 -> LO1/2
  RO1/1 -> LO1/1
  single2/1 -> RO2/2, RO1/2
  single1/1 -> RO2/1, RO1/1
  In1/1 -> single1/1
  In2/1 -> single2/1

== system lib_all/SubSystem518/SubSystem523/SubSystem577/SubSystem580 ==
  note: Text82
  note: Text81
  Block1298 : Inport
  Block1299 : Inport  Port=2
  Block1300 : Inport  Port=3
  Block1301 : Constant  Value=720  OutDataTypeStr=double  SampleTime=-1
  Block1302 : Constant  Value=0  OutDataTypeStr=double  SampleTime=-1
  Block1303 : Constant  Value=0  OutDataTypeStr=double  SampleTime=-1
  Block1304 : Constant  Value=5  OutDataTypeStr=double  SampleTime=-1
  Block1305 : DataStoreRead  DataStoreName=DSM50
  Block1306 : DataStoreRead  DataStoreName=DSM35
  Block1307 : Gain  Gain=5  SaturateOnIntegerOverflow=on  OutDataTypeStr=Inherit: Same as input  ParamDataTypeStr=Inherit: Same as input
      settings: AttributesFormatString=Attribute283
  Block1308 : Gain  Gain=5  SaturateOnIntegerOverflow=on  OutDataTypeStr=Inherit: Same as input  ParamDataTypeStr=Inherit: Same as input
      settings: AttributesFormatString=Attribute284
  Block1309 : Gain  Gain=1/5  SaturateOnIntegerOverflow=on  OutDataTypeStr=Inherit: Same as input  ParamDataTypeStr=Inherit: Same as input
      settings: AttributesFormatString=Attribute285
  Block1310 : Product  Inputs=*/  OutDataTypeStr=double  SaturateOnIntegerOverflow=on  RndMeth=Zero  InputSameDT=on
  Block1311 : Rounding
  Block1312 : Rounding
  Block1313 : Sum  Inputs=+-+-  OutDataTypeStr=double  RndMeth=Zero  SaturateOnIntegerOverflow=on  InputSameDT=on
  Block1314 : Sum  Inputs=-+  OutDataTypeStr=double  RndMeth=Zero  SaturateOnIntegerOverflow=on  InputSameDT=on
  SubSystem581 : Subsystem  TreatAsAtomicUnit=on  mask(***ATC.DIVISION***){bd_version=1.01.A, r0=チェック有り, r1=, r2=, r3=, r4=, r5=, r6=, r7=, r8=, r9=, c0=, c1=, c2=, c3=, c4=, c5=, c6=, c7=, c8=, c9=}
      mask prompts: bd_version "Version"
      mask help: これはAutoCからのブロックです．編集禁止．
      settings: OpenFcn=warndlg('変更禁止!');
      mask Help: web(ui_blockhelpmng(gcb));
  SubSystem582 : Subsystem  TreatAsAtomicUnit=on  mask(***ATC.DSRVEC***){bd_version=1.01.A, r0=DSM95, r1=-1, r2=, r3=, r4=, r5=, r6=, r7=, r8=, r9=, c0=, c1=, c2=, c3=, c4=, c5=, c6=, c7=, c8=, c9=}
      mask prompts: bd_version "Version"
      mask help: これはAutoCからのブロックです．編集禁止．
      settings: OpenFcn=warndlg('変更禁止!');
      mask Help: web(ui_blockhelpmng(gcb));
      mask Display: disp('ATC.DSRVEC')
  SubSystem583 : Subsystem
      settings: RTWSystemCode=Inline
  Block1323 : Outport
  Block1324 : Outport  Port=2
  wires:
  Block1306/1 -> SubSystem581/2(div)
  Block1314/1 -> Block1324/1
  Block1307/1 -> Block1314/1
  Block1311/1 -> Block1307/1
  Block1310/1 -> Block1311/1
  Block1304/1 -> Block1310/2
  Block1299/1 -> SubSystem582/1(element)
  Block1303/1 -> SubSystem583/3(Block1317)
  Block1313/1 -> SubSystem583/2(Block1316)  "SigLabel30"
  Block1301/1 -> SubSystem583/1(Block1315)
  Block1300/1 -> Block1313/4
  SubSystem583/1(Block1322) -> Block1310/1, Block1314/2, Block1309/1
  Block1298/1 -> Block1308/1
  SubSystem581/1(Out) -> Block1313/3  "SigLabel29"
  Block1302/1 -> SubSystem581/3(init)
  SubSystem582/1(Output) -> SubSystem581/1(mult)
  Block1305/1 -> Block1313/2
  Block1308/1 -> Block1313/1
  Block1309/1 -> Block1312/1
  Block1312/1 -> Block1323/1

== system lib_all/SubSystem518/SubSystem523/SubSystem577/SubSystem586 ==
  note: Text85
  note: Text84
  note: Text83
  Block1325 : Inport
  Block1326 : Inport  Port=2
  Block1327 : Inport  Port=3
  Block1328 : Constant  Value=0  OutDataTypeStr=double  SampleTime=-1
  Block1329 : Constant  OutDataTypeStr=double  SampleTime=-1
  Block1330 : Constant  OutDataTypeStr=double  SampleTime=-1
  Block1331 : Constant  Value=0  OutDataTypeStr=double  SampleTime=-1
  Block1332 : Constant  Value=2  OutDataTypeStr=double  SampleTime=-1
  Block1333 : Constant  OutDataTypeStr=double  SampleTime=-1
  Block1334 : Constant  Value=143  OutDataTypeStr=double  SampleTime=-1
  Block1335 : Demux  Outputs=2
  Block1336 : Logic  Operator=NOT
  Block1337 : Mux  Inputs=2
  Block1338 : Mux  Inputs=2
  Block1339 : Sum  OutDataTypeStr=double  RndMeth=Zero  SaturateOnIntegerOverflow=on  InputSameDT=on
  Block1340 : Sum  OutDataTypeStr=double  RndMeth=Zero  SaturateOnIntegerOverflow=on  InputSameDT=on
  Block{1341..1344} (4 alike) : Switch  Threshold=0.5  RndMeth=Zero  SaturateOnIntegerOverflow=on  InputSameDT=on
  SubSystem587 : Subsystem
  SubSystem588 : Subsystem
  SubSystem589 : Subsystem
  SubSystem590 : Subsystem
  SubSystem591 : Subsystem  TreatAsAtomicUnit=on
  SubSystem600 : Subsystem  TreatAsAtomicUnit=on
  SubSystem610 : Subsystem  TreatAsAtomicUnit=on
  SubSystem620 : Subsystem  TreatAsAtomicUnit=on
  Block1404 : Outport
  Block1405 : Outport  Port=2
  wires:
  Block1327/1 -> SubSystem620/2(Block1390), SubSystem610/2(Block1375), SubSystem600/2(Block1360), SubSystem591/2(Block1346)
  Block1344/1 -> Block1342/3
  SubSystem620/1(Block1403) -> Block1344/3
  SubSystem610/1(Block1388) -> Block1344/1
  Block1336/1 -> SubSystem620/Enable
  SubSystem590/1(Out1) -> Block1344/2, SubSystem610/Enable, Block1336/1
  Block1332/1 -> SubSystem590/2(In2)
  Block1337/1 -> Block1343/1
  Block1339/1 -> Block1337/2
  Block1338/1 -> Block1343/3
  Block1331/1 -> Block1338/2
  Block1330/1 -> Block1339/1
  SubSystem587/1(Out1) -> Block1343/2
  Block1341/1 -> Block1335/1
  Block1335/2 -> Block1405/1
  Block1335/1 -> Block1404/1
  Block1340/1 -> SubSystem620/1(Block1389), SubSystem610/1(Block1374), SubSystem600/1(Block1359), SubSystem591/1(Block1345)
  Block1342/1 -> Block1341/3
  SubSystem600/1(Block1373) -> Block1342/1
  SubSystem589/1(Out1) -> SubSystem600/Enable, Block1342/2
  Block1329/1 -> SubSystem589/2(In2)
  SubSystem591/1(Block1358) -> Block1341/1
  SubSystem588/1(Out1) -> SubSystem591/Enable, Block1341/2
  Block1328/1 -> SubSystem588/2(In2)
  Block1326/1 -> SubSystem590/1(In1), SubSystem589/1(In1), SubSystem588/1(In1)
  Block1334/1 -> Block1338/1, SubSystem587/2(In2)
  Block1325/1 -> Block1337/1, Block1339/2, SubSystem587/1(In1)
  Block1333/1 -> Block1340/1
  Block1343/1 -> Block1340/2

== system lib_all/SubSystem660/SubSystem673/SubSystem676/SubSystem677 ==
  note: ***編集禁止***
  In1 : Inport
  In2 : Inport  Port=2
  RO1 : RelationalOperator  Operator===  InputSameDT=on
  single1 : DataTypeConversion  OutDataTypeStr=single  SaturateOnIntegerOverflow=on  RndMeth=Zero
  single2 : DataTypeConversion  OutDataTypeStr=single  SaturateOnIntegerOverflow=on  RndMeth=Zero
  Out1 : Outport
  wires:
  single2/1 -> RO1/2
  single1/1 -> RO1/1
  In1/1 -> single1/1
  In2/1 -> single2/1
  RO1/1 -> Out1/1

== system lib_all/SubSystem660/SubSystem673/SubSystem676/SubSystem678 ==
  note: ***編集禁止***
  In1 : Inport
  In2 : Inport  Port=2
  RO1 : RelationalOperator  Operator===  InputSameDT=on
  single1 : DataTypeConversion  OutDataTypeStr=single  SaturateOnIntegerOverflow=on  RndMeth=Zero
  single2 : DataTypeConversion  OutDataTypeStr=single  SaturateOnIntegerOverflow=on  RndMeth=Zero
  Out1 : Outport
  wires:
  single2/1 -> RO1/2
  single1/1 -> RO1/1
  In1/1 -> single1/1
  In2/1 -> single2/1
  RO1/1 -> Out1/1

== system lib_all/SubSystem660/SubSystem673/SubSystem676/SubSystem679 ==
  Block1529 : Inport
  Block1530 : Inport  Port=2
  Block1531 : Inport  Port=3
  Block1532 : Sum  OutDataTypeStr=double  RndMeth=Zero  SaturateOnIntegerOverflow=on  InputSameDT=on
  Block1533 : Switch  Threshold=0.5  RndMeth=Zero  SaturateOnIntegerOverflow=on  InputSameDT=on
  SubSystem680 : Subsystem
  Block1534 : Outport
  wires:
  Block1530/1 -> SubSystem680/1(In1), Block1533/3, Block1532/2
  Block1531/1 -> SubSystem680/2(In2)
  SubSystem680/1(Out1) -> Block1533/2
  Block1532/1 -> Block1533/1
  Block1533/1 -> Block1534/1
  Block1529/1 -> Block1532/1

== system lib_all/SubSystem660/SubSystem673/SubSystem676/SubSystem681 ==
  Block1535 : Inport
  Block1536 : Inport  Port=2
  Block1537 : Inport  Port=3
  Block1538 : Sum  OutDataTypeStr=double  RndMeth=Zero  SaturateOnIntegerOverflow=on  InputSameDT=on
  Block1539 : Switch  Threshold=0.5  RndMeth=Zero  SaturateOnIntegerOverflow=on  InputSameDT=on
  SubSystem682 : Subsystem
  Block1540 : Outport
  wires:
  Block1536/1 -> SubSystem682/1(In1), Block1539/3, Block1538/2
  Block1537/1 -> SubSystem682/2(In2)
  SubSystem682/1(Out1) -> Block1539/2
  Block1538/1 -> Block1539/1
  Block1539/1 -> Block1540/1
  Block1535/1 -> Block1538/1

== system lib_all/SubSystem660/SubSystem673/SubSystem684/SubSystem685 ==
  note: ***編集禁止***
  In1 : Inport
  In2 : Inport  Port=2
  RO1 : RelationalOperator  Operator=>  InputSameDT=on
  single1 : DataTypeConversion  OutDataTypeStr=single  SaturateOnIntegerOverflow=on  RndMeth=Zero
  single2 : DataTypeConversion  OutDataTypeStr=single  SaturateOnIntegerOverflow=on  RndMeth=Zero
  Out1 : Outport
  wires:
  single2/1 -> RO1/2
  single1/1 -> RO1/1
  In1/1 -> single1/1
  In2/1 -> single2/1
  RO1/1 -> Out1/1

== system lib_all/SubSystem660/SubSystem686/SubSystem688/SubSystem689 ==
  note: ***編集禁止***
  mult : Inport
  div : Inport  Port=2
  init : Inport  Port=3
  Product : Subsystem  TreatAsAtomicUnit=on
      settings: RTWSystemCode=Inline
  Out : Outport
  wires:
  Product/1(Out) -> Out/1
  mult/1 -> Product/1(mult)
  div/1 -> Product/2(div)
  init/1 -> Product/3(init)

== system lib_all/SubSystem660/SubSystem686/SubSystem688/SubSystem690 ==
  note: ***編集禁止***
  element : Inport
  Inside_DSR : DataStoreRead  DataStoreName=DSM95
  Selector : Selector  IndexOptions=Index vector (port)  Indices=[1 3]  OutputSizes=1
  Output : Outport
  wires:
  Selector/1 -> Output/1
  element/1 -> Selector/2
  Inside_DSR/1 -> Selector/1

== system lib_all/SubSystem660/SubSystem686/SubSystem688/SubSystem691 ==
  Block1579 : Inport
  Block1580 : Inport  Port=2
  Block1581 : Inport  Port=3
  Block1582 : Sum  OutDataTypeStr=double  RndMeth=Zero  SaturateOnIntegerOverflow=on  InputSameDT=on
  Block1583 : Sum  Inputs=+-  OutDataTypeStr=double  RndMeth=Zero  SaturateOnIntegerOverflow=on  InputSameDT=on
  Block1584 : Switch  Threshold=0.5  RndMeth=Zero  SaturateOnIntegerOverflow=on  InputSameDT=on
  Block1585 : Switch  Threshold=0.5  RndMeth=Zero  SaturateOnIntegerOverflow=on  InputSameDT=on
  SubSystem692 : Subsystem
  SubSystem693 : Subsystem
  Block1586 : Outport
  wires:
  Block1580/1 -> SubSystem692/1(In1), Block1583/1, SubSystem693/1(In1), Block1585/3, Block1582/2
  Block1579/1 -> Block1582/1, Block1583/2, SubSystem692/2(In2)
  SubSystem692/1(Out1) -> Block1584/2
  Block1581/1 -> SubSystem693/2(In2)
  SubSystem693/1(Out1) -> Block1585/2
  Block1585/1 -> Block1584/3
  Block1582/1 -> Block1585/1
  Block1584/1 -> Block1586/1
  Block1583/1 -> Block1584/1

== system lib_all/SubSystem660/SubSystem686/SubSystem694/SubSystem695 ==
  note: ***編集禁止***
  In1 : Inport
  In2 : Inport  Port=2
  LO1 : Logic
  RO1 : RelationalOperator  Operator=<  InputSameDT=on
  RO2 : RelationalOperator  Operator=~=  InputSameDT=on
  single1 : DataTypeConversion  OutDataTypeStr=single  SaturateOnIntegerOverflow=on  RndMeth=Zero
  single2 : DataTypeConversion  OutDataTypeStr=single  SaturateOnIntegerOverflow=on  RndMeth=Zero
  Out1 : Outport
  wires:
  LO1/1 -> Out1/1
  RO2/1 -> LO1/2
  RO1/1 -> LO1/1
  In2/1 -> single2/1
  In1/1 -> single1/1
  single1/1 -> RO2/1, RO1/1
  single2/1 -> RO2/2, RO1/2

== system lib_all/SubSystem660/SubSystem686/SubSystem694/SubSystem696 ==
  note: ***編集禁止***
  In1 : Inport
  In2 : Inport  Port=2
  RO1 : RelationalOperator  Operator===  InputSameDT=on
  single1 : DataTypeConversion  OutDataTypeStr=single  SaturateOnIntegerOverflow=on  RndMeth=Zero
  single2 : DataTypeConversion  OutDataTypeStr=single  SaturateOnIntegerOverflow=on  RndMeth=Zero
  Out1 : Outport
  wires:
  RO1/1 -> Out1/1
  In2/1 -> single2/1
  In1/1 -> single1/1
  single1/1 -> RO1/1
  single2/1 -> RO1/2

== system lib_all/SubSystem660/SubSystem686/SubSystem694/SubSystem697 ==
  note: ***編集禁止***
  In1 : Inport
  In2 : Inport  Port=2
  RO1 : RelationalOperator  Operator===  InputSameDT=on
  single1 : DataTypeConversion  OutDataTypeStr=single  SaturateOnIntegerOverflow=on  RndMeth=Zero
  single2 : DataTypeConversion  OutDataTypeStr=single  SaturateOnIntegerOverflow=on  RndMeth=Zero
  Out1 : Outport
  wires:
  RO1/1 -> Out1/1
  In2/1 -> single2/1
  In1/1 -> single1/1
  single1/1 -> RO1/1
  single2/1 -> RO1/2

== system lib_all/SubSystem660/SubSystem686/SubSystem694/SubSystem698 ==
  Block1607 : Inport
  Block1608 : Inport  Port=2
  Block1609 : EnablePort
  Block1610 : Demux  Outputs=2
  Block1611 : Mux  Inputs=2
  SubSystem699 : Subsystem
  SubSystem703 : Subsystem
  Block1620 : Outport
  wires:
  Block1607/1 -> Block1610/1
  Block1608/1 -> SubSystem703/1(Block1616), SubSystem699/1(Block1612)
  Block1610/1 -> SubSystem699/2(Block1613)
  Block1610/2 -> SubSystem703/2(Block1617)
  SubSystem699/1(Block1615) -> Block1611/1
  SubSystem703/1(Block1619) -> Block1611/2
  Block1611/1 -> Block1620/1

== system lib_all/SubSystem660/SubSystem686/SubSystem694/SubSystem707 ==
  Block1621 : Inport
  Block1622 : Inport  Port=2
  Block1623 : EnablePort
  Block1624 : Demux  Outputs=2
  Block1625 : Mux  Inputs=2
  Block1626 : Sum  OutDataTypeStr=double  RndMeth=Zero  SaturateOnIntegerOverflow=on  InputSameDT=on
  SubSystem708 : Subsystem  TreatAsAtomicUnit=on  mask(***ATC.DSRVEC***){bd_version=1.01.A, r0=DSM4, r1=-1, r2=, r3=, r4=, r5=, r6=, r7=, r8=, r9=, c0=, c1=, c2=, c3=, c4=, c5=, c6=, c7=, c8=, c9=}
      mask prompts: bd_version "Version"
      mask help: これはAutoCからのブロックです．編集禁止．
      settings: OpenFcn=warndlg('変更禁止!');
      mask Help: web(ui_blockhelpmng(gcb));
      mask Display: disp('ATC.DSRVEC')
  SubSystem709 : Subsystem
  SubSystem713 : Subsystem
  Block1635 : Outport
  wires:
  Block1624/2 -> SubSystem713/2(Block1632)
  Block1624/1 -> SubSystem709/2(Block1628)
  Block1621/1 -> Block1624/1
  SubSystem709/1(Block1630) -> Block1625/1
  SubSystem713/1(Block1634) -> Block1625/2
  Block1626/1 -> Block1635/1
  SubSystem708/1(Output) -> Block1626/1
  Block1625/1 -> Block1626/2
  Block1622/1 -> SubSystem713/1(Block1631), SubSystem709/1(Block1627), SubSystem708/1(element)

== system lib_all/SubSystem660/SubSystem686/SubSystem694/SubSystem717 ==
  Block1636 : Inport
  Block1637 : Inport  Port=2
  Block1638 : EnablePort
  Block1639 : Demux  Outputs=2
  Block1640 : Mux  Inputs=2
  Block1641 : Sum  OutDataTypeStr=double  RndMeth=Zero  SaturateOnIntegerOverflow=on  InputSameDT=on
  SubSystem718 : Subsystem  TreatAsAtomicUnit=on  mask(***ATC.DSRVEC***){bd_version=1.01.A, r0=DSM2, r1=-1, r2=, r3=, r4=, r5=, r6=, r7=, r8=, r9=, c0=, c1=, c2=, c3=, c4=, c5=, c6=, c7=, c8=, c9=}
      mask prompts: bd_version "Version"
      mask help: これはAutoCからのブロックです．編集禁止．
      settings: OpenFcn=warndlg('変更禁止!');
      mask Help: web(ui_blockhelpmng(gcb));
      mask Display: disp('ATC.DSRVEC')
  SubSystem719 : Subsystem
  SubSystem723 : Subsystem
  Block1650 : Outport
  wires:
  Block1636/1 -> Block1639/1
  Block1639/1 -> SubSystem719/2(Block1643)
  Block1639/2 -> SubSystem723/2(Block1647)
  SubSystem719/1(Block1645) -> Block1640/1
  SubSystem723/1(Block1649) -> Block1640/2
  Block1641/1 -> Block1650/1
  Block1640/1 -> Block1641/2
  Block1637/1 -> SubSystem723/1(Block1646), SubSystem719/1(Block1642), SubSystem718/1(element)
  SubSystem718/1(Output) -> Block1641/1

== system lib_all/SubSystem7/SubSystem134/SubSystem136/SubSystem137 ==
  note: ***編集禁止***
  N : Inport
  For_loop : Stateflow chart  TreatAsAtomicUnit=on
  action : Outport
  i : Outport  Port=2
  wires:
  For_loop/2(function_call) -> action/1
  N/1 -> For_loop/1(N_Loop)
  For_loop/1(idx) -> i/1
